simulink model "HvacSnsrTFbFrnt"  (R2022b)
settings: Solver=FixedStepDiscrete  StartTime=0.0  StopTime=10.0  FixedStep=0.2  RelTol=1e-3
(part 1 of 2)
settings Model: Creator=blue.ge  LastModifiedBy=blue.ge
settings DataIOCC: SaveFormat=StructureWithTime
settings OptimizationCC: DefaultParameterBehavior=Inlined  EnhancedBackFolding=on  PassReuseOutputArgsAs=Individual arguments  ZeroExternalMemoryAtStartup=off  ZeroInternalMemoryAtStartup=off  OptimizeBlockOrder=speed  DifferentSizesBufferReuse=on  OptimizationCustomize=off  EfficientTunableParamExpr=on
settings DebuggingCC: ParameterTunabilityLossMsg=error
settings ModelReferenceCC: ModelReferenceNumInstancesAllowed=Single
settings SFSimCC: MATLABDynamicMemAlloc=off
settings RTWCC: Description=AUTOSAR  SystemTargetFile=autosar.tlc  GenCodeOnly=on  TemplateMakefile=ert_default_tmf
settings CodeAppCC: ForceParamTrailComments=on  IgnoreCustomStorageClasses=off  OperatorAnnotations=on  SimulinkDataObjDesc=on  SFDataObjDesc=on  InsertBlockDesc=on
settings STFCustomTargetCC: TargetLangStandard=C99 (ISO)  MultiwordLength=256  ParMdlRefBuildCompliant=on  CompOptLevelCompliant=on  IncludeMdlTerminateFcn=off  CombineOutputUpdateFcns=on  SuppressErrorStatus=on  ERTCustomFileBanners=on  SupportNonFinite=off  SupportComplex=off  SupportContinuousTime=off  SupportNonInlinedSFcns=off  RemoveResetFunc=on  MATLABClassNameForMDSCustomization=Simulink.SoftwareTarget.ERTCustomization  AutosarCompliant=on  UseToolchainInfoCompliant=on  LookupTableObjectStructAxisOrder=2,1,3,4,...  LUTObjectStructOrderEvenSpacing=Size,Table,Breakpoints  SystemTargetFile=autosar.tlc
settings ERTTargetCC: TargetLangStandard=C99 (ISO)  ConcurrentExecutionCompliant=off  IncludeMdlTerminateFcn=off  SuppressErrorStatus=on  SupportNonFinite=off  SupportComplex=off  ModelStepFunctionPrototypeControlCompliant=off  CPPClassGenCompliant=off  AutosarCompliant=on  LookupTableObjectStructAxisOrder=2,1,3,4,...  LUTObjectStructOrderEvenSpacing=Size,Table,Breakpoints  InlinedParameterPlacement=NonHierarchical  ERTCustomFileTemplate=example_file_process.tlc

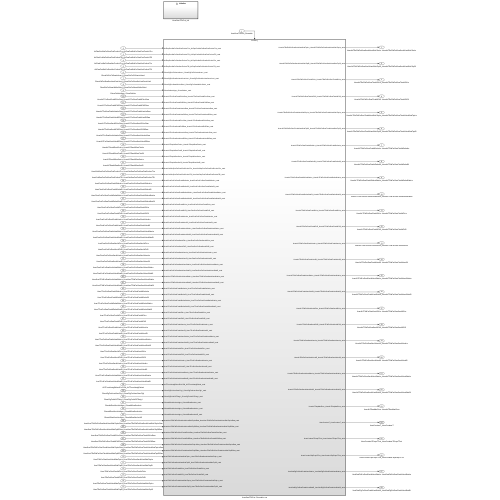
[thumbnail: Simulink's stored preview of the top-level diagram]
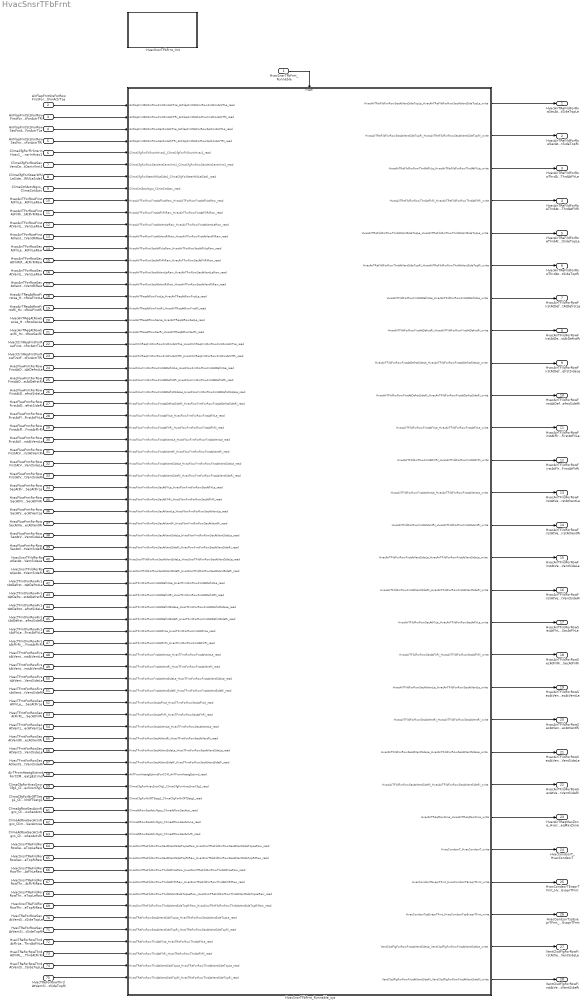
== system HvacSnsrTFbFrnt ==
  HvacSnsrTFbFrnt_Runnable : Inport  SampleTime=0.2  OutputFunctionCall=on
  AirFlapFrntStsForRowFirstForActrTLe_AirFlapFrntStsForRowFirstForActrTLe : Inport  Port=2  OutDataTypeStr=Enum: HvacActrSts  PortDimensions=1
  AirFlapFrntStsForRowFirstForActrTRi_AirFlapFrntStsForRowFirstForActrTRi : Inport  Port=3  OutDataTypeStr=Enum: HvacActrSts  PortDimensions=1
  AirFlapFrntStsForRowSecForActrTLe_AirFlapFrntStsForRowSecForActrTLe : Inport  Port=4  OutDataTypeStr=Enum: HvacActrSts  PortDimensions=1
  AirFlapFrntStsForRowSecForActrTRi_AirFlapFrntStsForRowSecForActrTRi : Inport  Port=5  OutDataTypeStr=Enum: HvacActrSts  PortDimensions=1
  ClimaCfgForFlrSnsrInHvac1_ClimaCfgForFlrSnsrInHvac1 : Inport  Port=6  OutDataTypeStr=Boolean  PortDimensions=1
  ClimaCfgForRowSecVentCentrVrnt1_ClimaCfgForRowSecVentCentrVrnt1 : Inport  Port=7  OutDataTypeStr=Enum: ClimaCfgForRowSecVentCentrVrnt1  PortDimensions=1
  ClimaCfgForSteerWhlLeSide1_ClimaCfgForSteerWhlLeSide1 : Inport  Port=8  OutDataTypeStr=Boolean  PortDimensions=1
  ClimaCtrlActvNgcc_ClimaCtrlActv : Inport  Port=9  OutDataTypeStr=Boolean  PortDimensions=1
  HvacAirTForRowFirstAtFlrLeRaw_HvacAirTForRowFirstAtFlrLeRaw : Inport  Port=10  OutDataTypeStr=Bus: HvacAirTForRowFirstAtFlrLe  PortDimensions=1  BusOutputAsStruct=on
  HvacAirTForRowFirstAtFlrRiRaw_HvacAirTForRowFirstAtFlrRiRaw : Inport  Port=11  OutDataTypeStr=Bus: HvacAirTForRowFirstAtFlrRi  PortDimensions=1  BusOutputAsStruct=on
  HvacAirTForRowFirstAtVentLeRaw_HvacAirTForRowFirstAtVentLeRaw : Inport  Port=12  OutDataTypeStr=Bus: HvacAirTForRowFirstAtVentLe  PortDimensions=1  BusOutputAsStruct=on
  HvacAirTForRowFirstAtVentRiRaw_HvacAirTForRowFirstAtVentRiRaw : Inport  Port=13  OutDataTypeStr=Bus: HvacAirTForRowFirstAtVentRi  PortDimensions=1  BusOutputAsStruct=on
  HvacAirTForRowSecAtFlrLeRaw_HvacAirTForRowSecAtFlrLeRaw : Inport  Port=14  OutDataTypeStr=Bus: HvacAirTForRowSecAtFlrLe  PortDimensions=1  BusOutputAsStruct=on
  HvacAirTForRowSecAtFlrRiRaw_HvacAirTForRowSecAtFlrRiRaw : Inport  Port=15  OutDataTypeStr=Bus: HvacAirTForRowSecAtFlrRi  PortDimensions=1  BusOutputAsStruct=on
  HvacAirTForRowSecAtVentLeRaw_HvacAirTForRowSecAtVentLeRaw : Inport  Port=16  OutDataTypeStr=Bus: HvacAirTForRowSecAtVentLe  PortDimensions=1  BusOutputAsStruct=on
  HvacAirTForRowSecAtVentRiRaw_HvacAirTForRowSecAtVentRiRaw : Inport  Port=17  OutDataTypeStr=Bus: HvacAirTForRowSecAtVentRi  PortDimensions=1  BusOutputAsStruct=on
  HvacAirTReqAtRowFirstLe_HvacAirTReqAtRowFirstLe : Inport  Port=18  OutDataTypeStr=T30  PortDimensions=1
  HvacAirTReqAtRowFirstRi_HvacAirTReqAtRowFirstRi : Inport  Port=19  OutDataTypeStr=T30  PortDimensions=1
  HvacAirTReqAtRowSecLe_HvacAirTReqAtRowSecLe : Inport  Port=20  OutDataTypeStr=T30  PortDimensions=1
  HvacAirTReqAtRowSecRi_HvacAirTReqAtRowSecRi : Inport  Port=21  OutDataTypeStr=T30  PortDimensions=1
  HvacCtrlrReqFrntForRowFirstForActrTLe_HvacCtrlrReqFrntForRowFirstForActrTLe : Inport  Port=22  OutDataTypeStr=Perc30  PortDimensions=1
  HvacCtrlrReqFrntForRowFirstForActrTRi_HvacCtrlrReqFrntForRowFirstForActrTRi : Inport  Port=23  OutDataTypeStr=Perc30  PortDimensions=1
  HvacFlowFrntForRowFirstAtDefrstLe_HvacFlowFrntForRowFirstAtDefrstLe : Inport  Port=24  OutDataTypeStr=AirFlow30  PortDimensions=1
  HvacFlowFrntForRowFirstAtDefrstRi_HvacFlowFrntForRowFirstAtDefrstRi : Inport  Port=25  OutDataTypeStr=AirFlow30  PortDimensions=1
  HvacFlowFrntForRowFirstAtDefrstSideLe_HvacFlowFrntForRowFirstAtDefrstSideLe : Inport  Port=26  OutDataTypeStr=AirFlow30  PortDimensions=1
  HvacFlowFrntForRowFirstAtDefrstSideRi_HvacFlowFrntForRowFirstAtDefrstSideRi : Inport  Port=27  OutDataTypeStr=AirFlow30  PortDimensions=1
  HvacFlowFrntForRowFirstAtFlrLe_HvacFlowFrntForRowFirstAtFlrLe : Inport  Port=28  OutDataTypeStr=AirFlow30  PortDimensions=1
  HvacFlowFrntForRowFirstAtFlrRi_HvacFlowFrntForRowFirstAtFlrRi : Inport  Port=29  OutDataTypeStr=AirFlow30  PortDimensions=1
  HvacFlowFrntForRowFirstAtVentLe_HvacFlowFrntForRowFirstAtVentLe : Inport  Port=30  OutDataTypeStr=AirFlow30  PortDimensions=1
  HvacFlowFrntForRowFirstAtVentRi_HvacFlowFrntForRowFirstAtVentRi : Inport  Port=31  OutDataTypeStr=AirFlow30  PortDimensions=1
  HvacFlowFrntForRowFirstAtVentSideLe_HvacFlowFrntForRowFirstAtVentSideLe : Inport  Port=32  OutDataTypeStr=AirFlow30  PortDimensions=1
  HvacFlowFrntForRowFirstAtVentSideRi_HvacFlowFrntForRowFirstAtVentSideRi : Inport  Port=33  OutDataTypeStr=AirFlow30  PortDimensions=1
  HvacFlowFrntForRowSecAtFlrLe_HvacFlowFrntForRowSecAtFlrLe : Inport  Port=34  OutDataTypeStr=AirFlow30  PortDimensions=1
  HvacFlowFrntForRowSecAtFlrRi_HvacFlowFrntForRowSecAtFlrRi : Inport  Port=35  OutDataTypeStr=AirFlow30  PortDimensions=1
  HvacFlowFrntForRowSecAtVentLe_HvacFlowFrntForRowSecAtVentLe : Inport  Port=36  OutDataTypeStr=AirFlow30  PortDimensions=1
  HvacFlowFrntForRowSecAtVentRi_HvacFlowFrntForRowSecAtVentRi : Inport  Port=37  OutDataTypeStr=AirFlow30  PortDimensions=1
  HvacFlowFrntForRowSecAtVentSideLe_HvacFlowFrntForRowSecAtVentSideLe : Inport  Port=38  OutDataTypeStr=AirFlow30  PortDimensions=1
  HvacFlowFrntForRowSecAtVentSideRi_HvacFlowFrntForRowSecAtVentSideRi : Inport  Port=39  OutDataTypeStr=AirFlow30  PortDimensions=1
  HvacSnsrTFildForRowSecAtVentSideLe_HvacSnsrTFildForRowSecAtVentSideLe : Inport  Port=40  OutDataTypeStr=Bus: HvacAirTForRowSecAtVentLe  PortDimensions=1  BusOutputAsStruct=on
  HvacSnsrTFildForRowSecAtVentSideRi_HvacSnsrTFildForRowSecAtVentSideRi : Inport  Port=41  OutDataTypeStr=Bus: HvacAirTForRowSecAtVentRi  PortDimensions=1  BusOutputAsStruct=on
  HvacTFrntForRowFirstAtDefrstLe_HvacTFrntForRowFirstAtDefrstLe : Inport  Port=42  OutDataTypeStr=T30  PortDimensions=1
  HvacTFrntForRowFirstAtDefrstRi_HvacTFrntForRowFirstAtDefrstRi : Inport  Port=43  OutDataTypeStr=T30  PortDimensions=1
  HvacTFrntForRowFirstAtDefrstSideLe_HvacTFrntForRowFirstAtDefrstSideLe : Inport  Port=44  OutDataTypeStr=T30  PortDimensions=1
  HvacTFrntForRowFirstAtDefrstSideRi_HvacTFrntForRowFirstAtDefrstSideRi : Inport  Port=45  OutDataTypeStr=T30  PortDimensions=1
  HvacTFrntForRowFirstAtFlrLe_HvacTFrntForRowFirstAtFlrLe : Inport  Port=46  OutDataTypeStr=T30  PortDimensions=1
  HvacTFrntForRowFirstAtFlrRi_HvacTFrntForRowFirstAtFlrRi : Inport  Port=47  OutDataTypeStr=T30  PortDimensions=1
  HvacTFrntForRowFirstAtVentLe_HvacTFrntForRowFirstAtVentLe : Inport  Port=48  OutDataTypeStr=T30  PortDimensions=1
  HvacTFrntForRowFirstAtVentRi_HvacTFrntForRowFirstAtVentRi : Inport  Port=49  OutDataTypeStr=T30  PortDimensions=1
  HvacTFrntForRowFirstAtVentSideLe_HvacTFrntForRowFirstAtVentSideLe : Inport  Port=50  OutDataTypeStr=T30  PortDimensions=1
  HvacTFrntForRowFirstAtVentSideRi_HvacTFrntForRowFirstAtVentSideRi : Inport  Port=51  OutDataTypeStr=T30  PortDimensions=1
  HvacTFrntForRowSecAtFlrLe_HvacTFrntForRowSecAtFlrLe : Inport  Port=52  OutDataTypeStr=T30  PortDimensions=1
  HvacTFrntForRowSecAtFlrRi_HvacTFrntForRowSecAtFlrRi : Inport  Port=53  OutDataTypeStr=T30  PortDimensions=1
  HvacTFrntForRowSecAtVentLe_HvacTFrntForRowSecAtVentLe : Inport  Port=54  OutDataTypeStr=T30  PortDimensions=1
  HvacTFrntForRowSecAtVentRi_HvacTFrntForRowSecAtVentRi : Inport  Port=55  OutDataTypeStr=T30  PortDimensions=1
  HvacTFrntForRowSecAtVentSideLe_HvacTFrntForRowSecAtVentSideLe : Inport  Port=56  OutDataTypeStr=T30  PortDimensions=1
  HvacTFrntForRowSecAtVentSideRi_HvacTFrntForRowSecAtVentSideRi : Inport  Port=57  OutDataTypeStr=T30  PortDimensions=1
  AirTFromHeatgEstimdForCCM_AirTFromHeatgEstimd : Inport  Port=58  OutDataTypeStr=T30  PortDimensions=1
  ClimaCfgForHvacSnsrCfg1_ClimaCfgForHvacSnsrCfg1 : Inport  Port=59  OutDataTypeStr=Bus: HvacSnsrCfg1  PortDimensions=1  BusOutputAsStruct=on
  ClimaCfgForNrOfTSetg1_ClimaCfgForNrOfTSetg1 : Inport  Port=60  OutDataTypeStr=Enum: ClimaCfgForNrOfTSetg1  PortDimensions=1
  ClimaAtRowSecActvNgcc_ClimaAtRowSecActv : Inport  Port=61  OutDataTypeStr=Boolean  PortDimensions=1
  ClimaAtRowSecActvNgcc_ClimaAtRowSecActvLe : Inport  Port=62  OutDataTypeStr=Boolean  PortDimensions=1
  ClimaAtRowSecActvNgcc_ClimaAtRowSecActvRi : Inport  Port=63  OutDataTypeStr=Boolean  PortDimensions=1
  HvacSnsrTReFildForRowSecAtVentSideTopLeRaw_HvacSnsrTReFildForRowSecAtVentSideTopLeRaw : Inport  Port=64  OutDataTypeStr=Bus: HvacAirTReForRowSecAtVentSideTopLe  PortDimensions=1  BusOutputAsStruct=on
  HvacSnsrTReFildForRowSecAtVentSideTopRiRaw_HvacSnsrTReFildForRowSecAtVentSideTopRiRaw : Inport  Port=65  OutDataTypeStr=Bus: HvacAirTReForRowSecAtVentSideTopRi  PortDimensions=1  BusOutputAsStruct=on
  HvacSnsrTReFildForRowThrdAtFlrLeRaw_HvacSnsrTReFildForRowThrdAtFlrLeRaw : Inport  Port=66  OutDataTypeStr=Bus: HvacAirTReForRowThrdAtFlrLe  PortDimensions=1  BusOutputAsStruct=on
  HvacSnsrTReFildForRowThrdAtFlrRiRaw_HvacSnsrTReFildForRowThrdAtFlrRiRaw : Inport  Port=67  OutDataTypeStr=Bus: HvacAirTReForRowThrdAtFlrRi  PortDimensions=1  BusOutputAsStruct=on
  HvacSnsrTReFildForRowThrdAtVentSideTopLeRaw_HvacSnsrTReFildForRowThrdAtVentSideTopLeRaw : Inport  Port=68  OutDataTypeStr=Bus: HvacAirTReForRowThrdAtVentSideTopLe  PortDimensions=1  BusOutputAsStruct=on
  HvacSnsrTReFildForRowThrdAtVentSideTopRiRaw_HvacSnsrTReFildForRowThrdAtVentSideTopRiRaw : Inport  Port=69  OutDataTypeStr=Bus: HvacAirTReForRowThrdAtVentSideTopRi  PortDimensions=1  BusOutputAsStruct=on
  HvacTReForRowSecAtVentSideTopLe_HvacTReForRowSecAtVentSideTopLe : Inport  Port=70  OutDataTypeStr=T30  PortDimensions=1
  HvacTReForRowSecAtVentSideTopRi_HvacTReForRowSecAtVentSideTopRi : Inport  Port=71  OutDataTypeStr=T30  PortDimensions=1
  HvacTReForRowThrdAtFlrLe_HvacTReForRowThrdAtFlrLe : Inport  Port=72  OutDataTypeStr=T30  PortDimensions=1
  HvacTReForRowThrdAtFlrRi_HvacTReForRowThrdAtFlrRi : Inport  Port=73  OutDataTypeStr=T30  PortDimensions=1
  HvacTReForRowThrdAtVentSideTopLe_HvacTReForRowThrdAtVentSideTopLe : Inport  Port=74  OutDataTypeStr=T30  PortDimensions=1
  HvacTReForRowThrdAtVentSideTopRi_HvacTReForRowThrdAtVentSideTopRi : Inport  Port=75  OutDataTypeStr=T30  PortDimensions=1
  HvacSnsrTFbFrnt_Init : Subsystem
  HvacSnsrTFbFrnt_Runnable_sys : Subsystem
  HvacAirTReFildForRowSecAtVentSideTopLe_HvacAirTReFildForRowSecAtVentSideTopLe : Outport  OutDataTypeStr=T30  PortDimensions=1
  HvacAirTReFildForRowSecAtVentSideTopRi_HvacAirTReFildForRowSecAtVentSideTopRi : Outport  Port=2  OutDataTypeStr=T30  PortDimensions=1
  HvacAirTReFildForRowThrdAtFlrLe_HvacAirTReFildForRowThrdAtFlrLe : Outport  Port=3  OutDataTypeStr=T30  PortDimensions=1
  HvacAirTReFildForRowThrdAtFlrRi_HvacAirTReFildForRowThrdAtFlrRi : Outport  Port=4  OutDataTypeStr=T30  PortDimensions=1
  HvacAirTReFildForRowThrdAtVentSideTopLe_HvacAirTReFildForRowThrdAtVentSideTopLe : Outport  Port=5  OutDataTypeStr=T30  PortDimensions=1
  HvacAirTReFildForRowThrdAtVentSideTopRi_HvacAirTReFildForRowThrdAtVentSideTopRi : Outport  Port=6  OutDataTypeStr=T30  PortDimensions=1
  HvacAirTFildForRowFirstAtDefrstLe_HvacAirTFildForRowFirstAtDefrstLe : Outport  Port=7  OutDataTypeStr=T30  PortDimensions=1
  HvacAirTFildForRowFirstAtDefrstRi_HvacAirTFildForRowFirstAtDefrstRi : Outport  Port=8  OutDataTypeStr=T30  PortDimensions=1
  HvacAirTFildForRowFirstAtDefrstSideLe_HvacAirTFildForRowFirstAtDefrstSideLe : Outport  Port=9  OutDataTypeStr=T30  PortDimensions=1
  HvacAirTFildForRowFirstAtDefrstSideRi_HvacAirTFildForRowFirstAtDefrstSideRi : Outport  Port=10  OutDataTypeStr=T30  PortDimensions=1
  HvacAirTFildForRowFirstAtFlrLe_HvacAirTFildForRowFirstAtFlrLe : Outport  Port=11  OutDataTypeStr=T30  PortDimensions=1
  HvacAirTFildForRowFirstAtFlrRi_HvacAirTFildForRowFirstAtFlrRi : Outport  Port=12  OutDataTypeStr=T30  PortDimensions=1
  HvacAirTFildForRowFirstAtVentLe_HvacAirTFildForRowFirstAtVentLe : Outport  Port=13  OutDataTypeStr=T30  PortDimensions=1
  HvacAirTFildForRowFirstAtVentRi_HvacAirTFildForRowFirstAtVentRi : Outport  Port=14  OutDataTypeStr=T30  PortDimensions=1
  HvacAirTFildForRowFirstAtVentSideLe_HvacAirTFildForRowFirstAtVentSideLe : Outport  Port=15  OutDataTypeStr=T30  PortDimensions=1
  HvacAirTFildForRowFirstAtVentSideRi_HvacAirTFildForRowFirstAtVentSideRi : Outport  Port=16  OutDataTypeStr=T30  PortDimensions=1
  HvacAirTFildForRowSecAtFlrLe_HvacAirTFildForRowSecAtFlrLe : Outport  Port=17  OutDataTypeStr=T30  PortDimensions=1
  HvacAirTFildForRowSecAtFlrRi_HvacAirTFildForRowSecAtFlrRi : Outport  Port=18  OutDataTypeStr=T30  PortDimensions=1
  HvacAirTFildForRowSecAtVentLe_HvacAirTFildForRowSecAtVentLe : Outport  Port=19  OutDataTypeStr=T30  PortDimensions=1
  HvacAirTFildForRowSecAtVentRi_HvacAirTFildForRowSecAtVentRi : Outport  Port=20  OutDataTypeStr=T30  PortDimensions=1
  HvacAirTFildForRowSecAtVentSideLe_HvacAirTFildForRowSecAtVentSideLe : Outport  Port=21  OutDataTypeStr=T30  PortDimensions=1
  HvacAirTFildForRowSecAtVentSideRi_HvacAirTFildForRowSecAtVentSideRi : Outport  Port=22  OutDataTypeStr=T30  PortDimensions=1
  HvacAirTReqMaxZone_HvacAirTReqMaxZone : Outport  Port=23  OutDataTypeStr=Enum: TempZone  PortDimensions=1
  HvacCondsorT_HvacCondsorT : Outport  Port=24  OutDataTypeStr=Bus: EvaprTFrnt  PortDimensions=1  BusOutputAsStruct=on
  HvacCondsorTEvaprTFrnt_HvacCondsorTEvaprTFrnt : Outport  Port=25  OutDataTypeStr=T30  PortDimensions=1
  HvacCondsorTspEvaprTFrnt_HvacCondsorTspEvaprTFrnt : Outport  Port=26  OutDataTypeStr=T30  PortDimensions=1
  VentClsdFlgForRowFirstAtVentSideLe_VentClsdFlgForRowFirstAtVentSideLe : Outport  Port=27  OutDataTypeStr=Boolean  PortDimensions=1
  VentClsdFlgForRowFirstAtVentSideRi_VentClsdFlgForRowFirstAtVentSideRi : Outport  Port=28  OutDataTypeStr=Boolean  PortDimensions=1
  wires:
  AirFlapFrntStsForRowFirstForActrTLe_AirFlapFrntStsForRowFirstForActrTLe/1 -> HvacSnsrTFbFrnt_Runnable_sys/1(AirFlapFrntStsForRowFirstForActrTLe_AirFlapFrntStsForRowFirstForActrTLe_read)
  AirFlapFrntStsForRowFirstForActrTRi_AirFlapFrntStsForRowFirstForActrTRi/1 -> HvacSnsrTFbFrnt_Runnable_sys/2(AirFlapFrntStsForRowFirstForActrTRi_AirFlapFrntStsForRowFirstForActrTRi_read)
  AirFlapFrntStsForRowSecForActrTLe_AirFlapFrntStsForRowSecForActrTLe/1 -> HvacSnsrTFbFrnt_Runnable_sys/3(AirFlapFrntStsForRowSecForActrTLe_AirFlapFrntStsForRowSecForActrTLe_read)
  AirFlapFrntStsForRowSecForActrTRi_AirFlapFrntStsForRowSecForActrTRi/1 -> HvacSnsrTFbFrnt_Runnable_sys/4(AirFlapFrntStsForRowSecForActrTRi_AirFlapFrntStsForRowSecForActrTRi_read)
  ClimaCfgForFlrSnsrInHvac1_ClimaCfgForFlrSnsrInHvac1/1 -> HvacSnsrTFbFrnt_Runnable_sys/5(ClimaCfgForFlrSnsrInHvac1_ClimaCfgForFlrSnsrInHvac1_read)
  ClimaCfgForRowSecVentCentrVrnt1_ClimaCfgForRowSecVentCentrVrnt1/1 -> HvacSnsrTFbFrnt_Runnable_sys/6(ClimaCfgForRowSecVentCentrVrnt1_ClimaCfgForRowSecVentCentrVrnt1_read)
  ClimaCfgForSteerWhlLeSide1_ClimaCfgForSteerWhlLeSide1/1 -> HvacSnsrTFbFrnt_Runnable_sys/7(ClimaCfgForSteerWhlLeSide1_ClimaCfgForSteerWhlLeSide1_read)
  ClimaCtrlActvNgcc_ClimaCtrlActv/1 -> HvacSnsrTFbFrnt_Runnable_sys/8(ClimaCtrlActvNgcc_ClimaCtrlActv_read)
  HvacAirTForRowFirstAtFlrLeRaw_HvacAirTForRowFirstAtFlrLeRaw/1 -> HvacSnsrTFbFrnt_Runnable_sys/9(HvacAirTForRowFirstAtFlrLeRaw_HvacAirTForRowFirstAtFlrLeRaw_read)
  HvacAirTForRowFirstAtFlrRiRaw_HvacAirTForRowFirstAtFlrRiRaw/1 -> HvacSnsrTFbFrnt_Runnable_sys/10(HvacAirTForRowFirstAtFlrRiRaw_HvacAirTForRowFirstAtFlrRiRaw_read)
  HvacAirTForRowFirstAtVentLeRaw_HvacAirTForRowFirstAtVentLeRaw/1 -> HvacSnsrTFbFrnt_Runnable_sys/11(HvacAirTForRowFirstAtVentLeRaw_HvacAirTForRowFirstAtVentLeRaw_read)
  HvacAirTForRowFirstAtVentRiRaw_HvacAirTForRowFirstAtVentRiRaw/1 -> HvacSnsrTFbFrnt_Runnable_sys/12(HvacAirTForRowFirstAtVentRiRaw_HvacAirTForRowFirstAtVentRiRaw_read)
  HvacAirTForRowSecAtFlrLeRaw_HvacAirTForRowSecAtFlrLeRaw/1 -> HvacSnsrTFbFrnt_Runnable_sys/13(HvacAirTForRowSecAtFlrLeRaw_HvacAirTForRowSecAtFlrLeRaw_read)
  HvacAirTForRowSecAtFlrRiRaw_HvacAirTForRowSecAtFlrRiRaw/1 -> HvacSnsrTFbFrnt_Runnable_sys/14(HvacAirTForRowSecAtFlrRiRaw_HvacAirTForRowSecAtFlrRiRaw_read)
  HvacAirTForRowSecAtVentLeRaw_HvacAirTForRowSecAtVentLeRaw/1 -> HvacSnsrTFbFrnt_Runnable_sys/15(HvacAirTForRowSecAtVentLeRaw_HvacAirTForRowSecAtVentLeRaw_read)
  HvacAirTForRowSecAtVentRiRaw_HvacAirTForRowSecAtVentRiRaw/1 -> HvacSnsrTFbFrnt_Runnable_sys/16(HvacAirTForRowSecAtVentRiRaw_HvacAirTForRowSecAtVentRiRaw_read)
  HvacAirTReqAtRowFirstLe_HvacAirTReqAtRowFirstLe/1 -> HvacSnsrTFbFrnt_Runnable_sys/17(HvacAirTReqAtRowFirstLe_HvacAirTReqAtRowFirstLe_read)
  HvacAirTReqAtRowFirstRi_HvacAirTReqAtRowFirstRi/1 -> HvacSnsrTFbFrnt_Runnable_sys/18(HvacAirTReqAtRowFirstRi_HvacAirTReqAtRowFirstRi_read)
  HvacAirTReqAtRowSecLe_HvacAirTReqAtRowSecLe/1 -> HvacSnsrTFbFrnt_Runnable_sys/19(HvacAirTReqAtRowSecLe_HvacAirTReqAtRowSecLe_read)
  HvacAirTReqAtRowSecRi_HvacAirTReqAtRowSecRi/1 -> HvacSnsrTFbFrnt_Runnable_sys/20(HvacAirTReqAtRowSecRi_HvacAirTReqAtRowSecRi_read)
  HvacCtrlrReqFrntForRowFirstForActrTLe_HvacCtrlrReqFrntForRowFirstForActrTLe/1 -> HvacSnsrTFbFrnt_Runnable_sys/21(HvacCtrlrReqFrntForRowFirstForActrTLe_HvacCtrlrReqFrntForRowFirstForActrTLe_read)
  HvacCtrlrReqFrntForRowFirstForActrTRi_HvacCtrlrReqFrntForRowFirstForActrTRi/1 -> HvacSnsrTFbFrnt_Runnable_sys/22(HvacCtrlrReqFrntForRowFirstForActrTRi_HvacCtrlrReqFrntForRowFirstForActrTRi_read)
  HvacFlowFrntForRowFirstAtDefrstLe_HvacFlowFrntForRowFirstAtDefrstLe/1 -> HvacSnsrTFbFrnt_Runnable_sys/23(HvacFlowFrntForRowFirstAtDefrstLe_HvacFlowFrntForRowFirstAtDefrstLe_read)
  HvacFlowFrntForRowFirstAtDefrstRi_HvacFlowFrntForRowFirstAtDefrstRi/1 -> HvacSnsrTFbFrnt_Runnable_sys/24(HvacFlowFrntForRowFirstAtDefrstRi_HvacFlowFrntForRowFirstAtDefrstRi_read)
  HvacFlowFrntForRowFirstAtDefrstSideLe_HvacFlowFrntForRowFirstAtDefrstSideLe/1 -> HvacSnsrTFbFrnt_Runnable_sys/25(HvacFlowFrntForRowFirstAtDefrstSideLe_HvacFlowFrntForRowFirstAtDefrstSideLe_read)
  HvacFlowFrntForRowFirstAtDefrstSideRi_HvacFlowFrntForRowFirstAtDefrstSideRi/1 -> HvacSnsrTFbFrnt_Runnable_sys/26(HvacFlowFrntForRowFirstAtDefrstSideRi_HvacFlowFrntForRowFirstAtDefrstSideRi_read)
  HvacFlowFrntForRowFirstAtFlrLe_HvacFlowFrntForRowFirstAtFlrLe/1 -> HvacSnsrTFbFrnt_Runnable_sys/27(HvacFlowFrntForRowFirstAtFlrLe_HvacFlowFrntForRowFirstAtFlrLe_read)
  HvacFlowFrntForRowFirstAtFlrRi_HvacFlowFrntForRowFirstAtFlrRi/1 -> HvacSnsrTFbFrnt_Runnable_sys/28(HvacFlowFrntForRowFirstAtFlrRi_HvacFlowFrntForRowFirstAtFlrRi_read)
  HvacFlowFrntForRowFirstAtVentLe_HvacFlowFrntForRowFirstAtVentLe/1 -> HvacSnsrTFbFrnt_Runnable_sys/29(HvacFlowFrntForRowFirstAtVentLe_HvacFlowFrntForRowFirstAtVentLe_read)
  HvacFlowFrntForRowFirstAtVentRi_HvacFlowFrntForRowFirstAtVentRi/1 -> HvacSnsrTFbFrnt_Runnable_sys/30(HvacFlowFrntForRowFirstAtVentRi_HvacFlowFrntForRowFirstAtVentRi_read)
  HvacFlowFrntForRowFirstAtVentSideLe_HvacFlowFrntForRowFirstAtVentSideLe/1 -> HvacSnsrTFbFrnt_Runnable_sys/31(HvacFlowFrntForRowFirstAtVentSideLe_HvacFlowFrntForRowFirstAtVentSideLe_read)
  HvacFlowFrntForRowFirstAtVentSideRi_HvacFlowFrntForRowFirstAtVentSideRi/1 -> HvacSnsrTFbFrnt_Runnable_sys/32(HvacFlowFrntForRowFirstAtVentSideRi_HvacFlowFrntForRowFirstAtVentSideRi_read)
  HvacFlowFrntForRowSecAtFlrLe_HvacFlowFrntForRowSecAtFlrLe/1 -> HvacSnsrTFbFrnt_Runnable_sys/33(HvacFlowFrntForRowSecAtFlrLe_HvacFlowFrntForRowSecAtFlrLe_read)
  HvacFlowFrntForRowSecAtFlrRi_HvacFlowFrntForRowSecAtFlrRi/1 -> HvacSnsrTFbFrnt_Runnable_sys/34(HvacFlowFrntForRowSecAtFlrRi_HvacFlowFrntForRowSecAtFlrRi_read)
  HvacFlowFrntForRowSecAtVentLe_HvacFlowFrntForRowSecAtVentLe/1 -> HvacSnsrTFbFrnt_Runnable_sys/35(HvacFlowFrntForRowSecAtVentLe_HvacFlowFrntForRowSecAtVentLe_read)
  HvacFlowFrntForRowSecAtVentRi_HvacFlowFrntForRowSecAtVentRi/1 -> HvacSnsrTFbFrnt_Runnable_sys/36(HvacFlowFrntForRowSecAtVentRi_HvacFlowFrntForRowSecAtVentRi_read)
  HvacFlowFrntForRowSecAtVentSideLe_HvacFlowFrntForRowSecAtVentSideLe/1 -> HvacSnsrTFbFrnt_Runnable_sys/37(HvacFlowFrntForRowSecAtVentSideLe_HvacFlowFrntForRowSecAtVentSideLe_read)
  HvacFlowFrntForRowSecAtVentSideRi_HvacFlowFrntForRowSecAtVentSideRi/1 -> HvacSnsrTFbFrnt_Runnable_sys/38(HvacFlowFrntForRowSecAtVentSideRi_HvacFlowFrntForRowSecAtVentSideRi_read)
  HvacSnsrTFildForRowSecAtVentSideLe_HvacSnsrTFildForRowSecAtVentSideLe/1 -> HvacSnsrTFbFrnt_Runnable_sys/39(HvacSnsrTFildForRowSecAtVentSideLe_HvacSnsrTFildForRowSecAtVentSideLe_read)
  HvacSnsrTFildForRowSecAtVentSideRi_HvacSnsrTFildForRowSecAtVentSideRi/1 -> HvacSnsrTFbFrnt_Runnable_sys/40(HvacSnsrTFildForRowSecAtVentSideRi_HvacSnsrTFildForRowSecAtVentSideRi_read)
  HvacTFrntForRowFirstAtDefrstLe_HvacTFrntForRowFirstAtDefrstLe/1 -> HvacSnsrTFbFrnt_Runnable_sys/41(HvacTFrntForRowFirstAtDefrstLe_HvacTFrntForRowFirstAtDefrstLe_read)
  HvacTFrntForRowFirstAtDefrstRi_HvacTFrntForRowFirstAtDefrstRi/1 -> HvacSnsrTFbFrnt_Runnable_sys/42(HvacTFrntForRowFirstAtDefrstRi_HvacTFrntForRowFirstAtDefrstRi_read)
  HvacTFrntForRowFirstAtDefrstSideLe_HvacTFrntForRowFirstAtDefrstSideLe/1 -> HvacSnsrTFbFrnt_Runnable_sys/43(HvacTFrntForRowFirstAtDefrstSideLe_HvacTFrntForRowFirstAtDefrstSideLe_read)
  HvacTFrntForRowFirstAtDefrstSideRi_HvacTFrntForRowFirstAtDefrstSideRi/1 -> HvacSnsrTFbFrnt_Runnable_sys/44(HvacTFrntForRowFirstAtDefrstSideRi_HvacTFrntForRowFirstAtDefrstSideRi_read)
  HvacTFrntForRowFirstAtFlrLe_HvacTFrntForRowFirstAtFlrLe/1 -> HvacSnsrTFbFrnt_Runnable_sys/45(HvacTFrntForRowFirstAtFlrLe_HvacTFrntForRowFirstAtFlrLe_read)
  HvacTFrntForRowFirstAtFlrRi_HvacTFrntForRowFirstAtFlrRi/1 -> HvacSnsrTFbFrnt_Runnable_sys/46(HvacTFrntForRowFirstAtFlrRi_HvacTFrntForRowFirstAtFlrRi_read)
  HvacTFrntForRowFirstAtVentLe_HvacTFrntForRowFirstAtVentLe/1 -> HvacSnsrTFbFrnt_Runnable_sys/47(HvacTFrntForRowFirstAtVentLe_HvacTFrntForRowFirstAtVentLe_read)
  HvacTFrntForRowFirstAtVentRi_HvacTFrntForRowFirstAtVentRi/1 -> HvacSnsrTFbFrnt_Runnable_sys/48(HvacTFrntForRowFirstAtVentRi_HvacTFrntForRowFirstAtVentRi_read)
  HvacTFrntForRowFirstAtVentSideLe_HvacTFrntForRowFirstAtVentSideLe/1 -> HvacSnsrTFbFrnt_Runnable_sys/49(HvacTFrntForRowFirstAtVentSideLe_HvacTFrntForRowFirstAtVentSideLe_read)
  HvacTFrntForRowFirstAtVentSideRi_HvacTFrntForRowFirstAtVentSideRi/1 -> HvacSnsrTFbFrnt_Runnable_sys/50(HvacTFrntForRowFirstAtVentSideRi_HvacTFrntForRowFirstAtVentSideRi_read)
  HvacTFrntForRowSecAtFlrLe_HvacTFrntForRowSecAtFlrLe/1 -> HvacSnsrTFbFrnt_Runnable_sys/51(HvacTFrntForRowSecAtFlrLe_HvacTFrntForRowSecAtFlrLe_read)
  HvacTFrntForRowSecAtFlrRi_HvacTFrntForRowSecAtFlrRi/1 -> HvacSnsrTFbFrnt_Runnable_sys/52(HvacTFrntForRowSecAtFlrRi_HvacTFrntForRowSecAtFlrRi_read)
  HvacTFrntForRowSecAtVentLe_HvacTFrntForRowSecAtVentLe/1 -> HvacSnsrTFbFrnt_Runnable_sys/53(HvacTFrntForRowSecAtVentLe_HvacTFrntForRowSecAtVentLe_read)
  HvacTFrntForRowSecAtVentRi_HvacTFrntForRowSecAtVentRi/1 -> HvacSnsrTFbFrnt_Runnable_sys/54(HvacTFrntForRowSecAtVentRi_HvacTFrntForRowSecAtVentRi_read)
  HvacTFrntForRowSecAtVentSideLe_HvacTFrntForRowSecAtVentSideLe/1 -> HvacSnsrTFbFrnt_Runnable_sys/55(HvacTFrntForRowSecAtVentSideLe_HvacTFrntForRowSecAtVentSideLe_read)
  HvacTFrntForRowSecAtVentSideRi_HvacTFrntForRowSecAtVentSideRi/1 -> HvacSnsrTFbFrnt_Runnable_sys/56(HvacTFrntForRowSecAtVentSideRi_HvacTFrntForRowSecAtVentSideRi_read)
  AirTFromHeatgEstimdForCCM_AirTFromHeatgEstimd/1 -> HvacSnsrTFbFrnt_Runnable_sys/57(AirTFromHeatgEstimdForCCM_AirTFromHeatgEstimd_read)
  ClimaCfgForHvacSnsrCfg1_ClimaCfgForHvacSnsrCfg1/1 -> HvacSnsrTFbFrnt_Runnable_sys/58(ClimaCfgForHvacSnsrCfg1_ClimaCfgForHvacSnsrCfg1_read)
  ClimaCfgForNrOfTSetg1_ClimaCfgForNrOfTSetg1/1 -> HvacSnsrTFbFrnt_Runnable_sys/59(ClimaCfgForNrOfTSetg1_ClimaCfgForNrOfTSetg1_read)
  ClimaAtRowSecActvNgcc_ClimaAtRowSecActv/1 -> HvacSnsrTFbFrnt_Runnable_sys/60(ClimaAtRowSecActvNgcc_ClimaAtRowSecActv_read)
  ClimaAtRowSecActvNgcc_ClimaAtRowSecActvLe/1 -> HvacSnsrTFbFrnt_Runnable_sys/61(ClimaAtRowSecActvNgcc_ClimaAtRowSecActvLe_read)
  ClimaAtRowSecActvNgcc_ClimaAtRowSecActvRi/1 -> HvacSnsrTFbFrnt_Runnable_sys/62(ClimaAtRowSecActvNgcc_ClimaAtRowSecActvRi_read)
  HvacSnsrTReFildForRowSecAtVentSideTopLeRaw_HvacSnsrTReFildForRowSecAtVentSideTopLeRaw/1 -> HvacSnsrTFbFrnt_Runnable_sys/63(HvacSnsrTReFildForRowSecAtVentSideTopLeRaw_HvacSnsrTReFildForRowSecAtVentSideTopLeRaw_read)
  HvacSnsrTReFildForRowSecAtVentSideTopRiRaw_HvacSnsrTReFildForRowSecAtVentSideTopRiRaw/1 -> HvacSnsrTFbFrnt_Runnable_sys/64(HvacSnsrTReFildForRowSecAtVentSideTopRiRaw_HvacSnsrTReFildForRowSecAtVentSideTopRiRaw_read)
  HvacSnsrTReFildForRowThrdAtFlrLeRaw_HvacSnsrTReFildForRowThrdAtFlrLeRaw/1 -> HvacSnsrTFbFrnt_Runnable_sys/65(HvacSnsrTReFildForRowThrdAtFlrLeRaw_HvacSnsrTReFildForRowThrdAtFlrLeRaw_read)
  HvacSnsrTReFildForRowThrdAtFlrRiRaw_HvacSnsrTReFildForRowThrdAtFlrRiRaw/1 -> HvacSnsrTFbFrnt_Runnable_sys/66(HvacSnsrTReFildForRowThrdAtFlrRiRaw_HvacSnsrTReFildForRowThrdAtFlrRiRaw_read)
  HvacSnsrTReFildForRowThrdAtVentSideTopLeRaw_HvacSnsrTReFildForRowThrdAtVentSideTopLeRaw/1 -> HvacSnsrTFbFrnt_Runnable_sys/67(HvacSnsrTReFildForRowThrdAtVentSideTopLeRaw_HvacSnsrTReFildForRowThrdAtVentSideTopLeRaw_read)
  HvacSnsrTReFildForRowThrdAtVentSideTopRiRaw_HvacSnsrTReFildForRowThrdAtVentSideTopRiRaw/1 -> HvacSnsrTFbFrnt_Runnable_sys/68(HvacSnsrTReFildForRowThrdAtVentSideTopRiRaw_HvacSnsrTReFildForRowThrdAtVentSideTopRiRaw_read)
  HvacTReForRowSecAtVentSideTopLe_HvacTReForRowSecAtVentSideTopLe/1 -> HvacSnsrTFbFrnt_Runnable_sys/69(HvacTReForRowSecAtVentSideTopLe_HvacTReForRowSecAtVentSideTopLe_read)
  HvacTReForRowSecAtVentSideTopRi_HvacTReForRowSecAtVentSideTopRi/1 -> HvacSnsrTFbFrnt_Runnable_sys/70(HvacTReForRowSecAtVentSideTopRi_HvacTReForRowSecAtVentSideTopRi_read)
  HvacTReForRowThrdAtFlrLe_HvacTReForRowThrdAtFlrLe/1 -> HvacSnsrTFbFrnt_Runnable_sys/71(HvacTReForRowThrdAtFlrLe_HvacTReForRowThrdAtFlrLe_read)
  HvacTReForRowThrdAtFlrRi_HvacTReForRowThrdAtFlrRi/1 -> HvacSnsrTFbFrnt_Runnable_sys/72(HvacTReForRowThrdAtFlrRi_HvacTReForRowThrdAtFlrRi_read)
  HvacTReForRowThrdAtVentSideTopLe_HvacTReForRowThrdAtVentSideTopLe/1 -> HvacSnsrTFbFrnt_Runnable_sys/73(HvacTReForRowThrdAtVentSideTopLe_HvacTReForRowThrdAtVentSideTopLe_read)
  HvacTReForRowThrdAtVentSideTopRi_HvacTReForRowThrdAtVentSideTopRi/1 -> HvacSnsrTFbFrnt_Runnable_sys/74(HvacTReForRowThrdAtVentSideTopRi_HvacTReForRowThrdAtVentSideTopRi_read)
  HvacSnsrTFbFrnt_Runnable_sys/1(HvacAirTReFildForRowSecAtVentSideTopLe_HvacAirTReFildForRowSecAtVentSideTopLe_write) -> HvacAirTReFildForRowSecAtVentSideTopLe_HvacAirTReFildForRowSecAtVentSideTopLe/1
  HvacSnsrTFbFrnt_Runnable_sys/2(HvacAirTReFildForRowSecAtVentSideTopRi_HvacAirTReFildForRowSecAtVentSideTopRi_write) -> HvacAirTReFildForRowSecAtVentSideTopRi_HvacAirTReFildForRowSecAtVentSideTopRi/1
  HvacSnsrTFbFrnt_Runnable_sys/3(HvacAirTReFildForRowThrdAtFlrLe_HvacAirTReFildForRowThrdAtFlrLe_write) -> HvacAirTReFildForRowThrdAtFlrLe_HvacAirTReFildForRowThrdAtFlrLe/1
  HvacSnsrTFbFrnt_Runnable_sys/4(HvacAirTReFildForRowThrdAtFlrRi_HvacAirTReFildForRowThrdAtFlrRi_write) -> HvacAirTReFildForRowThrdAtFlrRi_HvacAirTReFildForRowThrdAtFlrRi/1
  HvacSnsrTFbFrnt_Runnable_sys/5(HvacAirTReFildForRowThrdAtVentSideTopLe_HvacAirTReFildForRowThrdAtVentSideTopLe_write) -> HvacAirTReFildForRowThrdAtVentSideTopLe_HvacAirTReFildForRowThrdAtVentSideTopLe/1
  HvacSnsrTFbFrnt_Runnable_sys/6(HvacAirTReFildForRowThrdAtVentSideTopRi_HvacAirTReFildForRowThrdAtVentSideTopRi_write) -> HvacAirTReFildForRowThrdAtVentSideTopRi_HvacAirTReFildForRowThrdAtVentSideTopRi/1
  HvacSnsrTFbFrnt_Runnable_sys/7(HvacAirTFildForRowFirstAtDefrstLe_HvacAirTFildForRowFirstAtDefrstLe_write) -> HvacAirTFildForRowFirstAtDefrstLe_HvacAirTFildForRowFirstAtDefrstLe/1
  HvacSnsrTFbFrnt_Runnable_sys/8(HvacAirTFildForRowFirstAtDefrstRi_HvacAirTFildForRowFirstAtDefrstRi_write) -> HvacAirTFildForRowFirstAtDefrstRi_HvacAirTFildForRowFirstAtDefrstRi/1
  HvacSnsrTFbFrnt_Runnable_sys/9(HvacAirTFildForRowFirstAtDefrstSideLe_HvacAirTFildForRowFirstAtDefrstSideLe_write) -> HvacAirTFildForRowFirstAtDefrstSideLe_HvacAirTFildForRowFirstAtDefrstSideLe/1
  HvacSnsrTFbFrnt_Runnable_sys/10(HvacAirTFildForRowFirstAtDefrstSideRi_HvacAirTFildForRowFirstAtDefrstSideRi_write) -> HvacAirTFildForRowFirstAtDefrstSideRi_HvacAirTFildForRowFirstAtDefrstSideRi/1
  HvacSnsrTFbFrnt_Runnable_sys/11(HvacAirTFildForRowFirstAtFlrLe_HvacAirTFildForRowFirstAtFlrLe_write) -> HvacAirTFildForRowFirstAtFlrLe_HvacAirTFildForRowFirstAtFlrLe/1
  HvacSnsrTFbFrnt_Runnable_sys/12(HvacAirTFildForRowFirstAtFlrRi_HvacAirTFildForRowFirstAtFlrRi_write) -> HvacAirTFildForRowFirstAtFlrRi_HvacAirTFildForRowFirstAtFlrRi/1
  HvacSnsrTFbFrnt_Runnable_sys/13(HvacAirTFildForRowFirstAtVentLe_HvacAirTFildForRowFirstAtVentLe_write) -> HvacAirTFildForRowFirstAtVentLe_HvacAirTFildForRowFirstAtVentLe/1
  HvacSnsrTFbFrnt_Runnable_sys/14(HvacAirTFildForRowFirstAtVentRi_HvacAirTFildForRowFirstAtVentRi_write) -> HvacAirTFildForRowFirstAtVentRi_HvacAirTFildForRowFirstAtVentRi/1
  HvacSnsrTFbFrnt_Runnable_sys/15(HvacAirTFildForRowFirstAtVentSideLe_HvacAirTFildForRowFirstAtVentSideLe_write) -> HvacAirTFildForRowFirstAtVentSideLe_HvacAirTFildForRowFirstAtVentSideLe/1
  HvacSnsrTFbFrnt_Runnable_sys/16(HvacAirTFildForRowFirstAtVentSideRi_HvacAirTFildForRowFirstAtVentSideRi_write) -> HvacAirTFildForRowFirstAtVentSideRi_HvacAirTFildForRowFirstAtVentSideRi/1
  HvacSnsrTFbFrnt_Runnable_sys/17(HvacAirTFildForRowSecAtFlrLe_HvacAirTFildForRowSecAtFlrLe_write) -> HvacAirTFildForRowSecAtFlrLe_HvacAirTFildForRowSecAtFlrLe/1
  HvacSnsrTFbFrnt_Runnable_sys/18(HvacAirTFildForRowSecAtFlrRi_HvacAirTFildForRowSecAtFlrRi_write) -> HvacAirTFildForRowSecAtFlrRi_HvacAirTFildForRowSecAtFlrRi/1
  HvacSnsrTFbFrnt_Runnable_sys/19(HvacAirTFildForRowSecAtVentLe_HvacAirTFildForRowSecAtVentLe_write) -> HvacAirTFildForRowSecAtVentLe_HvacAirTFildForRowSecAtVentLe/1
  HvacSnsrTFbFrnt_Runnable_sys/20(HvacAirTFildForRowSecAtVentRi_HvacAirTFildForRowSecAtVentRi_write) -> HvacAirTFildForRowSecAtVentRi_HvacAirTFildForRowSecAtVentRi/1
  HvacSnsrTFbFrnt_Runnable_sys/21(HvacAirTFildForRowSecAtVentSideLe_HvacAirTFildForRowSecAtVentSideLe_write) -> HvacAirTFildForRowSecAtVentSideLe_HvacAirTFildForRowSecAtVentSideLe/1
  HvacSnsrTFbFrnt_Runnable_sys/22(HvacAirTFildForRowSecAtVentSideRi_HvacAirTFildForRowSecAtVentSideRi_write) -> HvacAirTFildForRowSecAtVentSideRi_HvacAirTFildForRowSecAtVentSideRi/1
  HvacSnsrTFbFrnt_Runnable_sys/23(HvacAirTReqMaxZone_HvacAirTReqMaxZone_write) -> HvacAirTReqMaxZone_HvacAirTReqMaxZone/1
  HvacSnsrTFbFrnt_Runnable_sys/24(HvacCondsorT_HvacCondsorT_write) -> HvacCondsorT_HvacCondsorT/1
  HvacSnsrTFbFrnt_Runnable_sys/25(HvacCondsorTEvaprTFrnt_HvacCondsorTEvaprTFrnt_write) -> HvacCondsorTEvaprTFrnt_HvacCondsorTEvaprTFrnt/1
  HvacSnsrTFbFrnt_Runnable_sys/26(HvacCondsorTspEvaprTFrnt_HvacCondsorTspEvaprTFrnt_write) -> HvacCondsorTspEvaprTFrnt_HvacCondsorTspEvaprTFrnt/1
  HvacSnsrTFbFrnt_Runnable_sys/27(VentClsdFlgForRowFirstAtVentSideLe_VentClsdFlgForRowFirstAtVentSideLe_write) -> VentClsdFlgForRowFirstAtVentSideLe_VentClsdFlgForRowFirstAtVentSideLe/1
  HvacSnsrTFbFrnt_Runnable_sys/28(VentClsdFlgForRowFirstAtVentSideRi_VentClsdFlgForRowFirstAtVentSideRi_write) -> VentClsdFlgForRowFirstAtVentSideRi_VentClsdFlgForRowFirstAtVentSideRi/1
  HvacSnsrTFbFrnt_Runnable/1 -> HvacSnsrTFbFrnt_Runnable_sys/Trigger

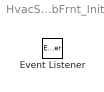
== system HvacSnsrTFbFrnt/HvacSnsrTFbFrnt_Init ==
  Event Listener : EventListener  EventName=reset
